simulink model "vlbipfb2_m3_roach"  (Simulink 7.3)
settings: Solver=ode45  StartTime=0.0  StopTime=1000  RelTol=1e-3
undefined symbols (not defined in the file or in its folder): pfb_coeff_gen_calc
libraries: xbsIndex_r4 x3204, casper_library x320, xbsReplacements_r4 x15, xps_library x13, vlbi_lib x9, simulink x4, inter_fir_lib x2
(part 1 of 34)
settings Model: Creator=rprimian  LastModifiedBy=rprimian
settings OptimizationCC: InitFltsAndDblsToZero=on
settings DebuggingCC: SaveWithParameterizedLinksMsg=none  SignalResolutionControl=TryResolveAllWithWarning  MultiTaskDSMMsg=warning  MultiTaskCondExecSysMsg=none  StrictBusMsg=None
settings RTWCC: IncludeERTFirstTime=on
settings GRTTargetCC: TargetFcnLib=ansi_tfl_tmw.mat

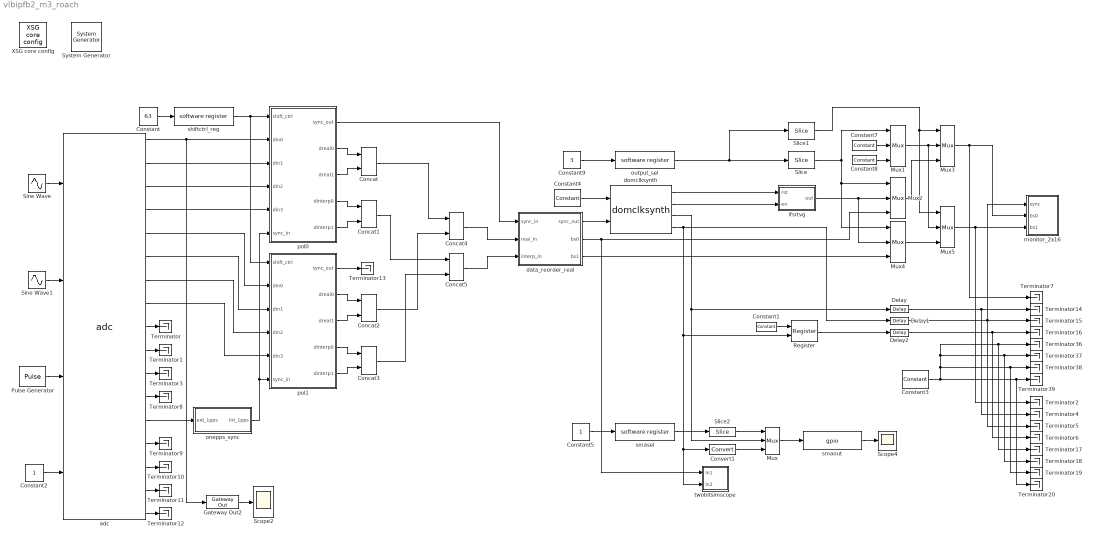
== system vlbipfb2_m3_roach ==
  " System Generator" : xbsIndex_r4/ System Generator  Tag=genX  SystemSampleTime=-1  xilinxfamily=virtex5  part=xc5vsx95t  speed=-1  package=ff1136  synthesis_tool=XST  clock_wrapper=Clock Enables  directory=./vlbipfb2_m3_roach/sysgen  testbench=off  simulink_period=1  sysclk_period=3.9062  dcm_input_clock_period=10  incr_netlist=off  trim_vbits=Everywhere in SubSystem  dbl_ovrd=According to Block Masks  core_generation=According to Block Masks  run_coregen=off  deprecated_control=off  eval_field=0  block_type=sysgen
      settings: block_version=11.3
  Concat{,1..5} (6 alike) : xbsIndex_r4/Concat  num_inputs=2  dbl_ovrd=off  block_type=concat
      settings: block_version=11.3
  Constant : Constant  Value=63  OutDataType=sfix(16)  OutScaling=2^0
  Constant1 : xbsIndex_r4/Constant  arith_type=Unsigned  const=1  n_bits=1  bin_pt=0  explicit_period=on  period=1  equ=P=C  opselect=C  inp2=PCIN>>17  opr=+  inp1=P  carry=CIN  dbl_ovrd=off  block_type=constant
      settings: block_version=11.3
  Constant2 : Constant  OutDataType=sfix(16)  OutScaling=2^0  SampleTime=1
  Constant3 : xbsIndex_r4/Constant  arith_type=Unsigned  const=0  n_bits=1  bin_pt=0  explicit_period=on  period=1  equ=P=C  opselect=C  inp2=PCIN>>17  opr=+  inp1=P  carry=CIN  dbl_ovrd=off  block_type=constant
      settings: block_version=11.3
  Constant4 : xbsIndex_r4/Constant  arith_type=Unsigned  const=6  n_bits=32  bin_pt=0  explicit_period=on  period=1  equ=P=C  opselect=C  inp2=PCIN>>17  opr=+  inp1=P  carry=CIN  dbl_ovrd=off  block_type=constant
      settings: block_version=11.3
  Constant5 : Constant  OutDataType=sfix(16)  OutScaling=2^0
  Constant7 : xbsIndex_r4/Constant  arith_type=Unsigned  const=0  n_bits=32  bin_pt=0  explicit_period=on  period=1  equ=P=C  opselect=C  inp2=PCIN>>17  opr=+  inp1=P  carry=CIN  dbl_ovrd=off  block_type=constant
      settings: block_version=11.3
  Constant8 : xbsIndex_r4/Constant  arith_type=Unsigned  const=2^32-1  n_bits=32  bin_pt=0  explicit_period=on  period=1  equ=P=C  opselect=C  inp2=PCIN>>17  opr=+  inp1=P  carry=CIN  dbl_ovrd=off  block_type=constant
      settings: block_version=11.3
  Constant9 : Constant  Value=3  OutDataType=sfix(16)  OutScaling=2^0
  Convert1 : xbsIndex_r4/Convert  arith_type=Unsigned  n_bits=1  bin_pt=0  quantization=Truncate  overflow=Wrap  latency=0  dbl_ovrd=off  pipeline=off  block_type=convert
      settings: xl_area=[0, 0, 0, 0, 0, 0, 0]  block_version=11.3
  Delay : xbsIndex_r4/Delay  en=off  latency=1  dbl_ovrd=off  reg_retiming=off  block_type=delay
      settings: xl_area=[0, 0, 0, 0, 0, 0, 0]  block_version=11.3
  Delay1 : xbsIndex_r4/Delay  en=off  latency=1  dbl_ovrd=off  reg_retiming=off  block_type=delay
      settings: xl_area=[0, 0, 0, 0, 0, 0, 0]  block_version=11.3
  Delay2 : xbsIndex_r4/Delay  en=off  latency=0  dbl_ovrd=off  reg_retiming=off  block_type=delay
      settings: xl_area=[0, 0, 0, 0, 0, 0, 0]  block_version=11.3
  Gateway Out2 : xbsIndex_r4/Gateway Out  hdl_port=off  timing_constraint=None  locs_specified=off  LOCs={}  block_type=gatewayout
      settings: infoedit="Gateway out block.  Converts Xilinx fixed point inputs into ouputs of type Simulink integer, double, or fixed point.<br><br>Hardware notes:  In hardware these blocks become top level output ports or are discarded, depending on how they are configured."  xl_area=[0, 0, 0, 0, 0, 0, 0]  block_version=11.3
  Mux : xbsIndex_r4/Mux  inputs=2  en=off  latency=0  precision=Full  arith_type="Signed  (2's comp)"  n_bits=8  bin_pt=2  quantization=Truncate  overflow=Wrap  dbl_ovrd=off  block_type=mux
      settings: xl_area=[0, 0, 0, 0, 0, 0, 0]  block_version=11.3
  Mux{1..5} (5 alike) : xbsIndex_r4/Mux  inputs=2  en=off  latency=1  precision=Full  arith_type="Signed  (2's comp)"  n_bits=8  bin_pt=2  quantization=Truncate  overflow=Wrap  dbl_ovrd=off  block_type=mux
      settings: xl_area=[0, 0, 0, 0, 0, 0, 0]  block_version=11.3
  Pulse Generator : DiscretePulseGenerator  Amplitude=1  Period=2560  PulseWidth=1  PhaseDelay=20  SampleTime=1
  Register : xbsIndex_r4/Register  init=0  rst=off  en=on  dbl_ovrd=off  block_type=register
      settings: xl_area=[0, 0, 0, 0, 0, 0, 0]  block_version=11.3
  Scope2 : Scope
      settings: SaveName=ScopeData5  MaxDataPoints=10000
  Scope4 : Scope
      settings: TimeRange=94.87951807228916  YMin=0.5138408304498272  YMax=0.5276816608996542  SaveName=ScopeData8
  Sine Wave : Sin  Amplitude=.8  Bias=0  Frequency=2*pi*119/256  Phase=0  Samples=2*pi*129/1024  SampleTime=1/4  Offset=0
  Sine Wave1 : Sin  Amplitude=.8  Bias=0  Frequency=2*pi*77/256  Phase=0  Samples=2*pi*129/1024  SampleTime=1/4  Offset=0
  Slice : xbsIndex_r4/Slice  nbits=1  boolean_output=off  mode=Lower Bit Location + Width  bit1=0  base1=MSB of Input  bit0=0  base0=LSB of Input  dbl_ovrd=off  block_type=slice
      settings: block_version=11.3
  Slice1 : xbsIndex_r4/Slice  nbits=1  boolean_output=off  mode=Lower Bit Location + Width  bit1=0  base1=MSB of Input  bit0=1  base0=LSB of Input  dbl_ovrd=off  block_type=slice
      settings: block_version=11.3
  Slice2 : xbsIndex_r4/Slice  nbits=1  boolean_output=off  mode=Lower Bit Location + Width  bit1=0  base1=MSB of Input  bit0=0  base0=LSB of Input  dbl_ovrd=off  block_type=slice
      settings: block_version=11.3
  Terminator{,1..20,36..39} (25 alike) : Terminator
  XSG core config : xps_library/XSG core config  Tag=xps:xsg  SystemSampleTime=-1  hw_sys=ROACH:sx95t  ibob_linux=off  clk_src=adc0_clk  iBOB_clk_src=sys_clk  BEE2_ctrl_clk_src=sys_clk  BEE2_usr_clk_src=sys_clk  ROACH_clk_src=adc0_clk  CORR_clk_src=sys_clk  gpio_clk_io_group=iBOB:gpio0  gpio_clk_bit_index=0  clk_rate=256  sample_period=1  synthesis_tool=XST  mpc_type=powerpc440_ext
  adc : xps_library/adc  Tag=xps:adc  SystemSampleTime=-1  adc_brd=adc0  adc_clk_rate=1024  adc_interleave=off  sample_period=1
  data_reorder_real : Subsystem  mask{sample_width=2, vsi_clock=64 MHz}
      mask prompts: sample_width "Channel sample width:"; vsi_clock "VSI output clock rate:"
      mask init:
      | myname = gcb;
      | 
      | if (strcmp(vsi_clock, '32 MHz'))
      |     set_param([gcb, '/restream'], 'clock_rate', '32');
      |     set_param([gcb, '/slc_wrmask'], 'bit1', '-1');
      | else
      |     set_param([gcb, '/restream'], 'clock_rate', '64');
      |     set_param([gcb, '/slc_wrmask'], 'bit1', '0');
      | end
      mask StyleString: edit,popup(32 MHz|64 MHz)
  domclksynth : vlbi_lib/domclksynth  SystemSampleTime=-1
  lfsrtvg : Subsystem
  monitor_2x16 : Subsystem  mask{sample_width=2}
      mask prompts: sample_width "Sample width"
  onepps_sync : Subsystem
  output_sel : xps_library/software register  Tag=xps:sw_reg  SystemSampleTime=-1  io_dir=From Processor  arith_type=Unsigned  bitwidth=32  bin_pt=0  sample_period=1  gw_name=vlbipfb2_m3_roach_output_sel_user_data_out
  pol0 : Subsystem
  pol1 : Subsystem
  shiftctrl_reg : xps_library/software register  Tag=xps:sw_reg  SystemSampleTime=-1  io_dir=From Processor  arith_type=Unsigned  bitwidth=32  bin_pt=0  sample_period=1  gw_name=vlbipfb2_m3_roach_shiftctrl_reg_user_data_out
  smaout : xps_library/gpio  Tag=xps:gpio  SystemSampleTime=-1  io_group=ROACH:led  io_dir=out  arith_type=Unsigned  bitwidth=1  bin_pt=0  bit_index=1  sample_period=1  use_single_ended=off  use_ddr=off  reg_iob=on  reg_clk_phase=0  termination=None
  smasel : xps_library/software register  Tag=xps:sw_reg  SystemSampleTime=-1  io_dir=From Processor  arith_type=Unsigned  bitwidth=32  bin_pt=0  sample_period=1  gw_name=vlbipfb2_m3_roach_smasel_user_data_out
  twobitsimscope : Subsystem
  wires:
  Constant/1 -> shiftctrl_reg/1
  adc/13 -> onepps_sync/1(ext_1pps)
  shiftctrl_reg/1 -> pol0/1(shift_ctrl), pol1/1(shift_ctrl)
  adc/1 -> Gateway Out2/1, pol0/2(din0)
  adc/2 -> pol0/3(din1)
  adc/3 -> pol0/4(din2)
  adc/4 -> pol0/5(din3)
  onepps_sync/1(int_1pps) -> pol0/6(sync_in), pol1/6(sync_in)
  domclksynth/2 -> lfsrtvg/2(en)
  Constant4/1 -> domclksynth/1
  domclksynth/3 -> Mux/2, Delay/1
  domclksynth/4 -> Register/2, Convert1/1, twobitsimscope/2(In2), Delay1/1
  Constant3/1 -> Terminator37/1, Terminator18/1, Terminator36/1, Terminator17/1, Terminator38/1, Terminator19/1, Terminator39/1, Terminator20/1
  Constant2/1 -> adc/4
  Pulse Generator/1 -> adc/3
  data_reorder_real/1(sync_out) -> domclksynth/2
  data_reorder_real/2(bs0) -> Mux2/3, twobitsimscope/1(In1)
  domclksynth/1 -> lfsrtvg/1(rst)
  Sine Wave/1 -> adc/1
  Constant1/1 -> Register/1
  Register/1 -> Delay2/1
  Gateway Out2/1 -> Scope2/1
  Mux/1 -> smaout/1
  smasel/1 -> Slice2/1
  Constant5/1 -> smasel/1
  Convert1/1 -> Mux/3
  Slice2/1 -> Mux/1
  smaout/1 -> Scope4/1
  output_sel/1 -> Slice/1, Slice1/1
  Constant9/1 -> output_sel/1
  Constant7/1 -> Mux1/2
  Constant8/1 -> Mux1/3
  Slice/1 -> Mux1/1, Mux2/1, Mux4/1
  lfsrtvg/1(out) -> Mux2/2, Mux4/2
  Mux1/1 -> Mux3/2, Mux5/2
  Mux2/1 -> Mux3/3
  Slice1/1 -> Mux3/1, Mux5/1
  Mux3/1 -> monitor_2x16/2(bs0), Terminator7/1
  Delay/1 -> Terminator14/1, Terminator4/1
  Delay1/1 -> monitor_2x16/1(sync), Terminator15/1, Terminator5/1
  Delay2/1 -> Terminator16/1, Terminator6/1
  adc/5 -> pol1/2(din0)
  adc/6 -> pol1/3(din1)
  adc/7 -> pol1/4(din2)
  adc/8 -> pol1/5(din3)
  adc/9 -> Terminator/1
  adc/10 -> Terminator1/1
  adc/11 -> Terminator3/1
  adc/12 -> Terminator8/1
  adc/14 -> Terminator9/1
  adc/15 -> Terminator10/1
  adc/16 -> Terminator11/1
  adc/17 -> Terminator12/1
  pol0/1(sync_out) -> data_reorder_real/1(sync_in)
  pol0/2(dreal0) -> Concat/1
  pol0/3(dreal1) -> Concat/2
  pol0/4(dinterp0) -> Concat1/1
  pol0/5(dinterp1) -> Concat1/2
  Concat4/1 -> data_reorder_real/2(real_in)
  Concat5/1 -> data_reorder_real/3(interp_in)
  Concat/1 -> Concat4/1
  Concat2/1 -> Concat4/2
  Concat1/1 -> Concat5/1
  Concat3/1 -> Concat5/2
  data_reorder_real/3(bs1) -> Mux4/3
  Mux4/1 -> Mux5/3
  Mux5/1 -> monitor_2x16/3(bs1), Terminator2/1
  pol1/1(sync_out) -> Terminator13/1
  pol1/2(dreal0) -> Concat2/1
  pol1/3(dreal1) -> Concat2/2
  pol1/4(dinterp0) -> Concat3/1
  pol1/5(dinterp1) -> Concat3/2
  Sine Wave1/1 -> adc/2

== system vlbipfb2_m3_roach/data_reorder_real ==
  sync_in : Inport  OutDataType=sfix(16)  OutScaling=2^0
  real_in : Inport  Port=2  OutDataType=sfix(16)  OutScaling=2^0
  interp_in : Inport  Port=3  OutDataType=sfix(16)  OutScaling=2^0
  Concat : xbsIndex_r4/Concat  num_inputs=4  dbl_ovrd=off  block_type=concat
      settings: block_version=11.3
  Concat1 : xbsIndex_r4/Concat  num_inputs=4  dbl_ovrd=off  block_type=concat
      settings: block_version=11.3
  Concat2 : xbsIndex_r4/Concat  num_inputs=2  dbl_ovrd=off  block_type=concat
      settings: block_version=11.3
  Constant : xbsIndex_r4/Constant  arith_type=Unsigned  const=0  n_bits=32*sample_width  bin_pt=0  explicit_period=on  period=1  equ=P=C  opselect=C  inp2=PCIN>>17  opr=+  inp1=P  carry=CIN  dbl_ovrd=off  block_type=constant
      settings: block_version=11.3
  Constant1 : xbsIndex_r4/Constant  arith_type=Boolean  const=0  n_bits=8*sample_width  bin_pt=0  explicit_period=on  period=1  equ=P=C  opselect=C  inp2=PCIN>>17  opr=+  inp1=P  carry=CIN  dbl_ovrd=off  block_type=constant
      settings: block_version=11.3
  Convert : xbsIndex_r4/Convert  arith_type=Boolean  n_bits=8  bin_pt=6  quantization=Truncate  overflow=Wrap  latency=0  dbl_ovrd=off  pipeline=off  block_type=convert
      settings: xl_area=[0, 0, 0, 0, 0, 0, 0]  block_version=11.3
  Counter : xbsIndex_r4/Counter  cnt_type=Free Running  cnt_to=Inf  operation=Up  start_count=0  cnt_by_val=1  arith_type=Unsigned  n_bits=1  bin_pt=0  load_pin=off  rst=on  en=off  explicit_period=off  period=1  dbl_ovrd=off  use_behavioral_HDL=off  implementation=Fabric  use_rpm=on  block_type=counter
      settings: xl_area=[0, 0, 0, 0, 0, 0, 0]  block_version=11.3
  Counter1 : xbsIndex_r4/Counter  cnt_type=Free Running  cnt_to=Inf  operation=Up  start_count=0  cnt_by_val=1  arith_type=Unsigned  n_bits=3  bin_pt=0  load_pin=off  rst=on  en=off  explicit_period=off  period=1  dbl_ovrd=off  use_behavioral_HDL=off  implementation=Fabric  use_rpm=on  block_type=counter
      settings: xl_area=[0, 0, 0, 0, 0, 0, 0]  block_version=11.3
  Counter2 : xbsIndex_r4/Counter  cnt_type=Free Running  cnt_to=Inf  operation=Up  start_count=0  cnt_by_val=1  arith_type=Unsigned  n_bits=1  bin_pt=0  load_pin=off  rst=on  en=off  explicit_period=off  period=1  dbl_ovrd=off  use_behavioral_HDL=off  implementation=Fabric  use_rpm=on  block_type=counter
      settings: xl_area=[0, 0, 0, 0, 0, 0, 0]  block_version=11.3
  Delay{,1..3} (4 alike) : xbsIndex_r4/Delay  en=off  latency=1  dbl_ovrd=off  reg_retiming=off  block_type=delay
      settings: xl_area=[0, 0, 0, 0, 0, 0, 0]  block_version=11.3
  Delay4 : xbsIndex_r4/Delay  en=off  latency=3  dbl_ovrd=off  reg_retiming=off  block_type=delay
      settings: xl_area=[0, 0, 0, 0, 0, 0, 0]  block_version=11.3
  Delay7 : xbsIndex_r4/Delay  en=off  latency=11  dbl_ovrd=off  reg_retiming=off  block_type=delay
      settings: xl_area=[0, 0, 0, 0, 0, 0, 0]  block_version=11.3
  Inverter : xbsIndex_r4/Inverter  en=off  latency=0  dbl_ovrd=off  block_type=inv
      settings: xl_area=[0, 0, 0, 0, 0, 0, 0]  block_version=11.3
  Mux : xbsIndex_r4/Mux  inputs=2  en=off  latency=1  precision=Full  arith_type="Signed  (2's comp)"  n_bits=8  bin_pt=2  quantization=Truncate  overflow=Wrap  dbl_ovrd=off  block_type=mux
      settings: xl_area=[0, 0, 0, 0, 0, 0, 0]  block_version=11.3
  Register : xbsIndex_r4/Register  init=0  rst=off  en=on  dbl_ovrd=off  block_type=register
      settings: xl_area=[0, 0, 0, 0, 0, 0, 0]  block_version=11.3
  Register1 : xbsIndex_r4/Register  init=0  rst=off  en=on  dbl_ovrd=off  block_type=register
      settings: xl_area=[0, 0, 0, 0, 0, 0, 0]  block_version=11.3
  Slice : xbsIndex_r4/Slice  nbits=1  boolean_output=off  mode=Lower Bit Location + Width  bit1=0  base1=MSB of Input  bit0=0  base0=LSB of Input  dbl_ovrd=off  block_type=slice
      settings: block_version=11.3
  Slice1 : xbsIndex_r4/Slice  nbits=2  boolean_output=off  mode=Upper Bit Location + Width  bit1=0  base1=MSB of Input  bit0=0  base0=LSB of Input  dbl_ovrd=off  block_type=slice
      settings: block_version=11.3
  Terminator : Terminator
  monitor_x32 : Subsystem
  monitor_x8 : Subsystem
  reorder_bram : xbsIndex_r4/Dual Port RAM  depth=8  initVector=zeros(2)  distributed_mem=Block RAM  init_a=0  init_b=0  rst_a=off  rst_b=off  en_a=off  en_b=off  latency=3  write_mode_A=Read Before Write  write_mode_B=Read Before Write  dbl_ovrd=off  optimize=Area  use_rpm=off  block_type=dpram
      settings: xl_area=[0, 0, 0, 0, 0, 0, 0]  block_version=11.3
  restream : Subsystem  mask{clock_rate=64}
      mask prompts: clock_rate "Clock rate (MHz)"
  slc_p0i : xbsIndex_r4/Slice  nbits=2*sample_width  boolean_output=off  mode=Upper Bit Location + Width  bit1=0  base1=MSB of Input  bit0=0  base0=LSB of Input  dbl_ovrd=off  block_type=slice
      settings: block_version=11.3
  slc_p0r : xbsIndex_r4/Slice  nbits=2*sample_width  boolean_output=off  mode=Upper Bit Location + Width  bit1=0  base1=MSB of Input  bit0=0  base0=LSB of Input  dbl_ovrd=off  block_type=slice
      settings: block_version=11.3
  slc_p1i : xbsIndex_r4/Slice  nbits=2*sample_width  boolean_output=off  mode=Upper Bit Location + Width  bit1=-2*sample_width  base1=MSB of Input  bit0=0  base0=LSB of Input  dbl_ovrd=off  block_type=slice
      settings: block_version=11.3
  slc_p1r : xbsIndex_r4/Slice  nbits=2*sample_width  boolean_output=off  mode=Upper Bit Location + Width  bit1=-2*sample_width  base1=MSB of Input  bit0=0  base0=LSB of Input  dbl_ovrd=off  block_type=slice
      settings: block_version=11.3
  slc_wrmask : xbsIndex_r4/Slice  nbits=1  boolean_output=on  mode=Upper Bit Location + Width  bit1=0  base1=MSB of Input  bit0=0  base0=LSB of Input  dbl_ovrd=off  block_type=slice
      settings: block_version=11.3
  wrmask_count : xbsIndex_r4/Counter  cnt_type=Count Limited  cnt_to=31  operation=Up  start_count=0  cnt_by_val=1  arith_type=Unsigned  n_bits=6  bin_pt=0  load_pin=off  rst=on  en=off  explicit_period=off  period=1  dbl_ovrd=off  use_behavioral_HDL=off  implementation=Fabric  use_rpm=on  block_type=counter
      settings: xl_area=[0, 0, 0, 0, 0, 0, 0]  block_version=11.3
  sync_out : Outport  OutDataType=sfix(16)  OutScaling=2^0
  bs0 : Outport  Port=2  OutDataType=sfix(16)  OutScaling=2^0
  bs1 : Outport  Port=3  OutDataType=sfix(16)  OutScaling=2^0
  wires:
  Inverter/1 -> reorder_bram/3
  wrmask_count/1 -> slc_wrmask/1
  restream/3(bs1) -> bs1/1
  restream/2(bs0) -> bs0/1
  restream/1(sync_out) -> sync_out/1
  reorder_bram/2 -> monitor_x32/1(In1), restream/2(din)
  Delay7/1 -> monitor_x32/2(Sync), restream/1(sync_in)
  Concat2/1 -> reorder_bram/1
  Counter1/1 -> Slice1/1, Slice/1
  Slice1/1 -> Concat2/2
  Slice/1 -> Concat2/1
  reorder_bram/1 -> Terminator/1
  Counter2/1 -> reorder_bram/4
  Delay4/1 -> wrmask_count/1, Counter2/1, monitor_x8/2(Sync), Delay7/1, Counter1/1
  slc_wrmask/1 -> Inverter/1
  Mux/1 -> reorder_bram/2, monitor_x8/1(In1)
  Constant1/1 -> reorder_bram/6
  Constant/1 -> reorder_bram/5
  Register1/1 -> Mux/3
  Register/1 -> Mux/2
  Convert/1 -> Register1/2, Register/2
  Counter/1 -> Mux/1, Convert/1
  sync_in/1 -> Delay4/1, Counter/1
  Concat1/1 -> Register1/1
  Concat/1 -> Register/1
  Delay3/1 -> Concat1/3
  Delay2/1 -> Concat1/1
  Delay1/1 -> Concat/3
  Delay/1 -> Concat/1
  slc_p1i/1 -> Concat1/4, Delay3/1
  slc_p0i/1 -> Concat1/2, Delay2/1
  slc_p1r/1 -> Concat/4, Delay1/1
  slc_p0r/1 -> Concat/2, Delay/1
  interp_in/1 -> slc_p1i/1, slc_p0i/1
  real_in/1 -> slc_p1r/1, slc_p0r/1

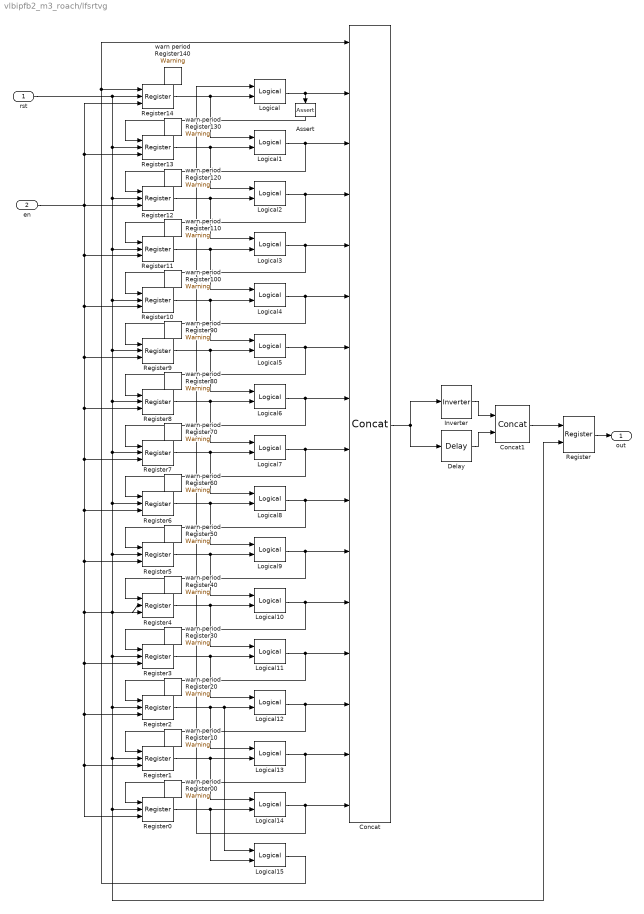
== system vlbipfb2_m3_roach/lfsrtvg ==
  rst : Inport  OutDataType=sfix(16)  OutScaling=2^0
  en : Inport  Port=2  OutDataType=sfix(16)  OutScaling=2^0
  Assert : xbsIndex_r4/Assert  assert_type=on  type_source=Explicitly  arith_type=Boolean  n_bits=16  bin_pt=14  assert_rate=on  rate_source=Explicitly  period=1  output_port=on  block_type=assert
      settings: block_version=11.3
  Concat : xbsIndex_r4/Concat  num_inputs=16  dbl_ovrd=off  block_type=concat
      settings: block_version=11.3
  Concat1 : xbsIndex_r4/Concat  num_inputs=2  dbl_ovrd=off  block_type=concat
      settings: block_version=11.3
  Delay : xbsIndex_r4/Delay  en=off  latency=0  dbl_ovrd=off  reg_retiming=off  block_type=delay
      settings: xl_area=[0, 0, 0, 0, 0, 0, 0]  block_version=11.3
  Inverter : xbsIndex_r4/Inverter  en=off  latency=0  dbl_ovrd=off  block_type=inv
      settings: xl_area=[0, 0, 0, 0, 0, 0, 0]  block_version=11.3
  Logical{,1..15} (16 alike) : xbsIndex_r4/Logical  logical_function=XOR  inputs=2  en=off  latency=0  precision=Full  arith_type=Unsigned  n_bits=8  bin_pt=2  align_bp=on  dbl_ovrd=off  block_type=logical
      settings: xl_area=[0, 0, 0, 0, 0, 0, 0]  block_version=11.3
  Register : xbsIndex_r4/Register  init=3221176320  rst=on  en=off  dbl_ovrd=off  block_type=register
      settings: xl_area=[0, 0, 0, 0, 0, 0, 0]  block_version=11.3
  Register{0..14} (15 alike) : xbsIndex_r4/Register  init=1  rst=on  en=on  dbl_ovrd=off  block_type=register
      settings: xl_area=[0, 0, 0, 0, 0, 0, 0]  block_version=11.3
  warn period Register00 : xbsReplacements_r4/Warning  SystemSampleTime=-1  period=1
  warn period Register{10,20,30,40,50,60,70,80,90,100,110,120,130,140} (14 alike) : xbsReplacements_r4/Warning  SystemSampleTime=-1  period=1
  out : Outport  OutDataType=sfix(16)  OutScaling=2^0
  wires:
  en/1 -> Register9/3, Register8/3, Register0/3, Register1/3, Register2/3, Register3/3, Register4/3, Register5/3, Register6/3, Register7/3, Register10/3, Register11/3, Register12/3, Register13/3, Register14/3
  rst/1 -> Register13/2, Register12/2, Register11/2, Register10/2, Register9/2, Register8/2, Register7/2, Register6/2, Register5/2, Register4/2, Register3/2, Register2/2, Register1/2, Register0/2, Register/2, Register14/2
  Register14/1 -> Logical1/1, Logical/2
  Register13/1 -> Logical2/1, Logical1/2
  Register12/1 -> Logical3/1, Logical2/2
  Register11/1 -> Logical4/1, Logical3/2
  Register10/1 -> Logical5/1, Logical4/2
  Register9/1 -> Logical6/1, Logical5/2
  Register8/1 -> Logical7/1, Logical6/2
  Register7/1 -> Logical8/1, Logical7/2
  Register6/1 -> Logical9/1, Logical8/2
  Register5/1 -> Logical10/1, Logical9/2
  Register4/1 -> Logical11/1, Logical10/2
  Register3/1 -> Logical12/1, Logical11/2
  Register2/1 -> Logical13/1, Logical12/2, Logical15/1
  Register1/1 -> Logical14/1, Logical13/2
  Register0/1 -> Logical14/2, Logical15/2
  Logical14/1 -> Logical/1, Concat/16
  Logical15/1 -> Register14/1, Concat/1
  Logical/1 -> Concat/2, Assert/1
  Logical1/1 -> Register12/1, Concat/3
  Logical2/1 -> Register11/1, Concat/4
  Logical3/1 -> Register10/1, Concat/5
  Logical4/1 -> Register9/1, Concat/6
  Logical5/1 -> Register8/1, Concat/7
  Logical6/1 -> Register7/1, Concat/8
  Logical7/1 -> Register6/1, Concat/9
  Logical8/1 -> Register5/1, Concat/10
  Logical9/1 -> Register4/1, Concat/11
  Logical10/1 -> Register3/1, Concat/12
  Logical11/1 -> Register2/1, Concat/13
  Logical12/1 -> Register1/1, Concat/14
  Logical13/1 -> Register0/1, Concat/15
  Concat/1 -> Inverter/1, Delay/1
  Inverter/1 -> Concat1/1
  Delay/1 -> Concat1/2
  Concat1/1 -> Register/1
  Register/1 -> out/1
  Assert/1 -> Register13/1

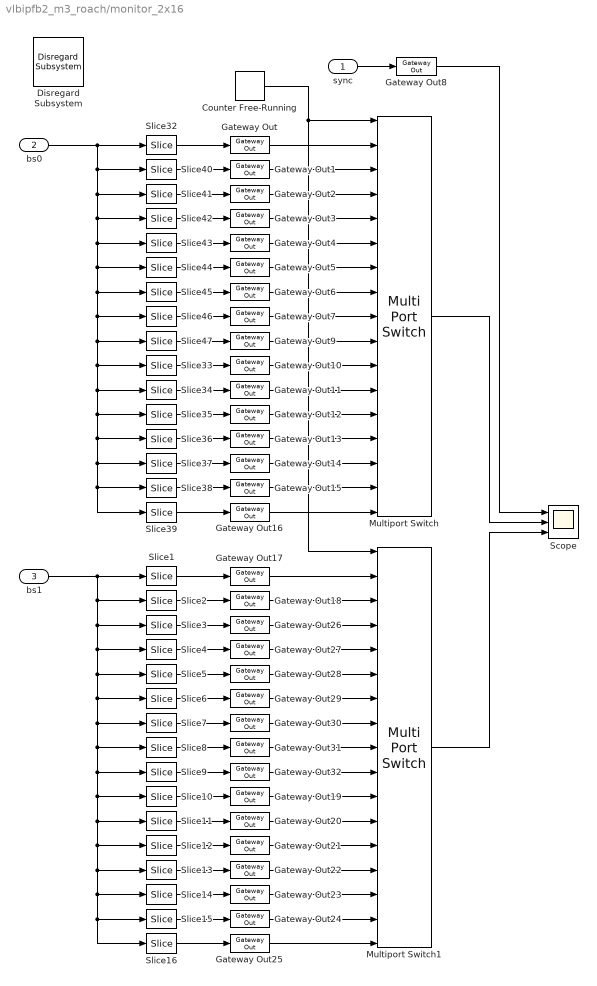
== system vlbipfb2_m3_roach/monitor_2x16 ==
  sync : Inport  OutDataType=sfix(16)  OutScaling=2^0
  bs0 : Inport  Port=2  OutDataType=sfix(16)  OutScaling=2^0
  bs1 : Inport  Port=3  OutDataType=sfix(16)  OutScaling=2^0
  Counter Free-Running : simulink/Sources/Counter Free-Running  SystemSampleTime=-1  NumBits=4  tsamp=1/16
  Disregard Subsystem : xbsIndex_r4/Disregard Subsystem  Tag=discardX  block_type=disregard
      settings: block_version=11.3
  Gateway Out{,1..32} (33 alike) : xbsIndex_r4/Gateway Out  hdl_port=off  timing_constraint=None  locs_specified=off  LOCs={}  block_type=gatewayout
      settings: infoedit="Gateway out block.  Converts Xilinx fixed point inputs into ouputs of type Simulink integer, double, or fixed point.<br><br>Hardware notes:  In hardware these blocks become top level output ports or are discarded, depending on how they are configured."  xl_area=[0, 0, 0, 0, 0, 0, 0]  block_version=11.3
  Multiport Switch : MultiPortSwitch  Inputs=16  zeroidx=on  SampleTime=1/16
  Multiport Switch1 : MultiPortSwitch  Inputs=16  zeroidx=on  SampleTime=1/16
  Scope : Scope  NumInputPorts=3
      settings: YMin=-5~-5~-5  YMax=5~5~5
  Slice1 : xbsIndex_r4/Slice  nbits=sample_width  boolean_output=off  mode=Upper Bit Location + Width  bit1=sample_width * 0  base1=MSB of Input  bit0=0  base0=LSB of Input  dbl_ovrd=off  block_type=slice
      settings: block_version=11.3
  Slice10 : xbsIndex_r4/Slice  nbits=sample_width  boolean_output=off  mode=Upper Bit Location + Width  bit1=sample_width * -9  base1=MSB of Input  bit0=0  base0=LSB of Input  dbl_ovrd=off  block_type=slice
      settings: block_version=11.3
  Slice11 : xbsIndex_r4/Slice  nbits=sample_width  boolean_output=off  mode=Upper Bit Location + Width  bit1=sample_width * -10  base1=MSB of Input  bit0=0  base0=LSB of Input  dbl_ovrd=off  block_type=slice
      settings: block_version=11.3
  Slice12 : xbsIndex_r4/Slice  nbits=sample_width  boolean_output=off  mode=Upper Bit Location + Width  bit1=sample_width * -11  base1=MSB of Input  bit0=0  base0=LSB of Input  dbl_ovrd=off  block_type=slice
      settings: block_version=11.3
  Slice13 : xbsIndex_r4/Slice  nbits=sample_width  boolean_output=off  mode=Upper Bit Location + Width  bit1=sample_width * -12  base1=MSB of Input  bit0=0  base0=LSB of Input  dbl_ovrd=off  block_type=slice
      settings: block_version=11.3
  Slice14 : xbsIndex_r4/Slice  nbits=sample_width  boolean_output=off  mode=Upper Bit Location + Width  bit1=sample_width * -13  base1=MSB of Input  bit0=0  base0=LSB of Input  dbl_ovrd=off  block_type=slice
      settings: block_version=11.3
  Slice15 : xbsIndex_r4/Slice  nbits=sample_width  boolean_output=off  mode=Upper Bit Location + Width  bit1=sample_width * -14  base1=MSB of Input  bit0=0  base0=LSB of Input  dbl_ovrd=off  block_type=slice
      settings: block_version=11.3
  Slice16 : xbsIndex_r4/Slice  nbits=sample_width  boolean_output=off  mode=Upper Bit Location + Width  bit1=sample_width * -15  base1=MSB of Input  bit0=0  base0=LSB of Input  dbl_ovrd=off  block_type=slice
      settings: block_version=11.3
  Slice2 : xbsIndex_r4/Slice  nbits=sample_width  boolean_output=off  mode=Upper Bit Location + Width  bit1=sample_width * -1  base1=MSB of Input  bit0=0  base0=LSB of Input  dbl_ovrd=off  block_type=slice
      settings: block_version=11.3
  Slice3 : xbsIndex_r4/Slice  nbits=sample_width  boolean_output=off  mode=Upper Bit Location + Width  bit1=sample_width * -2  base1=MSB of Input  bit0=0  base0=LSB of Input  dbl_ovrd=off  block_type=slice
      settings: block_version=11.3
  Slice32 : xbsIndex_r4/Slice  nbits=sample_width  boolean_output=off  mode=Upper Bit Location + Width  bit1=sample_width * 0  base1=MSB of Input  bit0=0  base0=LSB of Input  dbl_ovrd=off  block_type=slice
      settings: block_version=11.3
  Slice33 : xbsIndex_r4/Slice  nbits=sample_width  boolean_output=off  mode=Upper Bit Location + Width  bit1=sample_width * -9  base1=MSB of Input  bit0=0  base0=LSB of Input  dbl_ovrd=off  block_type=slice
      settings: block_version=11.3
  Slice34 : xbsIndex_r4/Slice  nbits=sample_width  boolean_output=off  mode=Upper Bit Location + Width  bit1=sample_width * -10  base1=MSB of Input  bit0=0  base0=LSB of Input  dbl_ovrd=off  block_type=slice
      settings: block_version=11.3
  Slice35 : xbsIndex_r4/Slice  nbits=sample_width  boolean_output=off  mode=Upper Bit Location + Width  bit1=sample_width * -11  base1=MSB of Input  bit0=0  base0=LSB of Input  dbl_ovrd=off  block_type=slice
      settings: block_version=11.3
  Slice36 : xbsIndex_r4/Slice  nbits=sample_width  boolean_output=off  mode=Upper Bit Location + Width  bit1=sample_width * -12  base1=MSB of Input  bit0=0  base0=LSB of Input  dbl_ovrd=off  block_type=slice
      settings: block_version=11.3
  Slice37 : xbsIndex_r4/Slice  nbits=sample_width  boolean_output=off  mode=Upper Bit Location + Width  bit1=sample_width * -13  base1=MSB of Input  bit0=0  base0=LSB of Input  dbl_ovrd=off  block_type=slice
      settings: block_version=11.3
  Slice38 : xbsIndex_r4/Slice  nbits=sample_width  boolean_output=off  mode=Upper Bit Location + Width  bit1=sample_width * -14  base1=MSB of Input  bit0=0  base0=LSB of Input  dbl_ovrd=off  block_type=slice
      settings: block_version=11.3
  Slice39 : xbsIndex_r4/Slice  nbits=sample_width  boolean_output=off  mode=Upper Bit Location + Width  bit1=sample_width * -15  base1=MSB of Input  bit0=0  base0=LSB of Input  dbl_ovrd=off  block_type=slice
      settings: block_version=11.3
  Slice4 : xbsIndex_r4/Slice  nbits=sample_width  boolean_output=off  mode=Upper Bit Location + Width  bit1=sample_width * -3  base1=MSB of Input  bit0=0  base0=LSB of Input  dbl_ovrd=off  block_type=slice
      settings: block_version=11.3
  Slice40 : xbsIndex_r4/Slice  nbits=sample_width  boolean_output=off  mode=Upper Bit Location + Width  bit1=sample_width * -1  base1=MSB of Input  bit0=0  base0=LSB of Input  dbl_ovrd=off  block_type=slice
      settings: block_version=11.3
  Slice41 : xbsIndex_r4/Slice  nbits=sample_width  boolean_output=off  mode=Upper Bit Location + Width  bit1=sample_width * -2  base1=MSB of Input  bit0=0  base0=LSB of Input  dbl_ovrd=off  block_type=slice
      settings: block_version=11.3
  Slice42 : xbsIndex_r4/Slice  nbits=sample_width  boolean_output=off  mode=Upper Bit Location + Width  bit1=sample_width * -3  base1=MSB of Input  bit0=0  base0=LSB of Input  dbl_ovrd=off  block_type=slice
      settings: block_version=11.3
  Slice43 : xbsIndex_r4/Slice  nbits=sample_width  boolean_output=off  mode=Upper Bit Location + Width  bit1=sample_width * -4  base1=MSB of Input  bit0=0  base0=LSB of Input  dbl_ovrd=off  block_type=slice
      settings: block_version=11.3
  Slice44 : xbsIndex_r4/Slice  nbits=sample_width  boolean_output=off  mode=Upper Bit Location + Width  bit1=sample_width * -5  base1=MSB of Input  bit0=0  base0=LSB of Input  dbl_ovrd=off  block_type=slice
      settings: block_version=11.3
  Slice45 : xbsIndex_r4/Slice  nbits=sample_width  boolean_output=off  mode=Upper Bit Location + Width  bit1=sample_width * -6  base1=MSB of Input  bit0=0  base0=LSB of Input  dbl_ovrd=off  block_type=slice
      settings: block_version=11.3
  Slice46 : xbsIndex_r4/Slice  nbits=sample_width  boolean_output=off  mode=Upper Bit Location + Width  bit1=sample_width * -7  base1=MSB of Input  bit0=0  base0=LSB of Input  dbl_ovrd=off  block_type=slice
      settings: block_version=11.3
  Slice47 : xbsIndex_r4/Slice  nbits=sample_width  boolean_output=off  mode=Upper Bit Location + Width  bit1=sample_width * -8  base1=MSB of Input  bit0=0  base0=LSB of Input  dbl_ovrd=off  block_type=slice
      settings: block_version=11.3
  Slice5 : xbsIndex_r4/Slice  nbits=sample_width  boolean_output=off  mode=Upper Bit Location + Width  bit1=sample_width * -4  base1=MSB of Input  bit0=0  base0=LSB of Input  dbl_ovrd=off  block_type=slice
      settings: block_version=11.3
  Slice6 : xbsIndex_r4/Slice  nbits=sample_width  boolean_output=off  mode=Upper Bit Location + Width  bit1=sample_width * -5  base1=MSB of Input  bit0=0  base0=LSB of Input  dbl_ovrd=off  block_type=slice
      settings: block_version=11.3
  Slice7 : xbsIndex_r4/Slice  nbits=sample_width  boolean_output=off  mode=Upper Bit Location + Width  bit1=sample_width * -6  base1=MSB of Input  bit0=0  base0=LSB of Input  dbl_ovrd=off  block_type=slice
      settings: block_version=11.3
  Slice8 : xbsIndex_r4/Slice  nbits=sample_width  boolean_output=off  mode=Upper Bit Location + Width  bit1=sample_width * -7  base1=MSB of Input  bit0=0  base0=LSB of Input  dbl_ovrd=off  block_type=slice
      settings: block_version=11.3
  Slice9 : xbsIndex_r4/Slice  nbits=sample_width  boolean_output=off  mode=Upper Bit Location + Width  bit1=sample_width * -8  base1=MSB of Input  bit0=0  base0=LSB of Input  dbl_ovrd=off  block_type=slice
      settings: block_version=11.3
  wires:
  bs1/1 -> Slice16/1, Slice15/1, Slice14/1, Slice13/1, Slice12/1, Slice11/1, Slice10/1, Slice9/1, Slice8/1, Slice7/1, Slice6/1, Slice5/1, Slice4/1, Slice3/1, Slice2/1, Slice1/1
  Multiport Switch1/1 -> Scope/3
  Multiport Switch/1 -> Scope/2
  Gateway Out8/1 -> Scope/1
  sync/1 -> Gateway Out8/1
  Gateway Out25/1 -> Multiport Switch1/17
  Gateway Out24/1 -> Multiport Switch1/16
  Gateway Out23/1 -> Multiport Switch1/15
  Gateway Out22/1 -> Multiport Switch1/14
  Gateway Out21/1 -> Multiport Switch1/13
  Gateway Out20/1 -> Multiport Switch1/12
  Gateway Out19/1 -> Multiport Switch1/11
  Gateway Out32/1 -> Multiport Switch1/10
  Gateway Out31/1 -> Multiport Switch1/9
  Gateway Out30/1 -> Multiport Switch1/8
  Gateway Out29/1 -> Multiport Switch1/7
  Gateway Out28/1 -> Multiport Switch1/6
  Gateway Out27/1 -> Multiport Switch1/5
  Gateway Out26/1 -> Multiport Switch1/4
  Gateway Out18/1 -> Multiport Switch1/3
  Gateway Out17/1 -> Multiport Switch1/2
  Gateway Out16/1 -> Multiport Switch/17
  Gateway Out15/1 -> Multiport Switch/16
  Gateway Out14/1 -> Multiport Switch/15
  Gateway Out13/1 -> Multiport Switch/14
  Gateway Out12/1 -> Multiport Switch/13
  Gateway Out11/1 -> Multiport Switch/12
  Gateway Out10/1 -> Multiport Switch/11
  Gateway Out9/1 -> Multiport Switch/10
  Gateway Out7/1 -> Multiport Switch/9
  Gateway Out6/1 -> Multiport Switch/8
  Gateway Out5/1 -> Multiport Switch/7
  Gateway Out4/1 -> Multiport Switch/6
  Gateway Out3/1 -> Multiport Switch/5
  Gateway Out2/1 -> Multiport Switch/4
  Gateway Out1/1 -> Multiport Switch/3
  Gateway Out/1 -> Multiport Switch/2
  Slice9/1 -> Gateway Out32/1
  Slice10/1 -> Gateway Out19/1
  Slice11/1 -> Gateway Out20/1
  Slice12/1 -> Gateway Out21/1
  Slice13/1 -> Gateway Out22/1
  Slice14/1 -> Gateway Out23/1
  Slice15/1 -> Gateway Out24/1
  Slice16/1 -> Gateway Out25/1
  Slice1/1 -> Gateway Out17/1
  Slice2/1 -> Gateway Out18/1
  Slice3/1 -> Gateway Out26/1
  Slice4/1 -> Gateway Out27/1
  Slice5/1 -> Gateway Out28/1
  Slice6/1 -> Gateway Out29/1
  Slice7/1 -> Gateway Out30/1
  Slice8/1 -> Gateway Out31/1
  Slice47/1 -> Gateway Out9/1
  Slice33/1 -> Gateway Out10/1
  Slice34/1 -> Gateway Out11/1
  Slice35/1 -> Gateway Out12/1
  Slice36/1 -> Gateway Out13/1
  Slice37/1 -> Gateway Out14/1
  Slice38/1 -> Gateway Out15/1
  Slice39/1 -> Gateway Out16/1
  bs0/1 -> Slice32/1, Slice40/1, Slice41/1, Slice42/1, Slice43/1, Slice44/1, Slice45/1, Slice46/1, Slice47/1, Slice33/1, Slice34/1, Slice35/1, Slice36/1, Slice37/1, Slice39/1, Slice38/1
  Slice32/1 -> Gateway Out/1
  Slice40/1 -> Gateway Out1/1
  Slice41/1 -> Gateway Out2/1
  Slice42/1 -> Gateway Out3/1
  Slice43/1 -> Gateway Out4/1
  Slice44/1 -> Gateway Out5/1
  Slice45/1 -> Gateway Out6/1
  Slice46/1 -> Gateway Out7/1
  Counter Free-Running/1 -> Multiport Switch1/1, Multiport Switch/1

== system vlbipfb2_m3_roach/onepps_sync ==
  ext_1pps : Inport  OutDataType=sfix(16)  OutScaling=2^0
  Step : Step  Time=200  Before=1  After=0  SampleTime=1
  Terminator : Terminator
  arm1pps : xps_library/software register  Tag=xps:sw_reg  SystemSampleTime=-1  io_dir=From Processor  arith_type=Unsigned  bitwidth=32  bin_pt=0  sample_period=1  gw_name=vlbipfb2_m3_roach_onepps_sync_arm1pps_user_data_out
  arm1pps_ack : xps_library/software register  Tag=xps:sw_reg  SystemSampleTime=-1  io_dir=To Processor  arith_type=Unsigned  bitwidth=32  bin_pt=0  sample_period=1  gw_name=vlbipfb2_m3_roach_onepps_sync_arm1pps_ack_user_data_in
  armshake : Subsystem
  int_1pps_gen : Subsystem
  int_1pps : Outport  OutDataType=sfix(16)  OutScaling=2^0
  wires:
  int_1pps_gen/1(1pps) -> int_1pps/1
  ext_1pps/1 -> armshake/1(ext_1pps)
  arm1pps/1 -> armshake/2(ext_arm1pps)
  Step/1 -> arm1pps/1
  armshake/1(rst_1pps) -> int_1pps_gen/1(rst)
  armshake/2(arm_ack) -> arm1pps_ack/1
  arm1pps_ack/1 -> Terminator/1
